simulink library "lib_all"  (Simulink 7.6)
settings: Solver=ode45  SolverMode=SingleTasking  StartTime=0.0  StopTime=10.0  RelTol=1e-3
values from neighbouring files (symbols this model uses):
  map_lib_03 = [6.8679 7.0159 7.4571 8.1849 9.1892 10.456 11.97 13.71 15.655 17.781 20.063 22.474 ... (803 elements, 73x11)]    [scr_simdata.mat]    (also in scr_simdata_all.mat)
  map_lib_06 = [2.5784 2.6727 2.9588 3.4453 4.1444 5.068 6.226 7.6242 9.2634 11.139 13.24 15.552 ... (803 elements, 73x11)]    [scr_simdata.mat]    (also in scr_simdata_all.mat)
  map_lib_09 = [0.0025784 0.0026727 0.0029588 0.0034453 0.0041444 0.005068 0.006226 0.0076242 0.0092634 0.011139 0.01324 0.015552 ... (803 elements, 73x11)]    [scr_simdata.mat]    (also in scr_simdata_all.mat)
  map_lib_10 = [2.74 2.75 2.76 2.77 2.78 2.79 2.8 2.81 2.82 2.83 2.84 2.85 ... (81 elements, 1x81)]    [scr_simdata.mat]    (also in scr_simdata_all.mat)
  map_lib_11 = [1.1983 1.2027 1.207 1.2113 1.2156 1.2198 1.2241 1.2283 1.2326 1.2368 1.241 1.2451 ... (81 elements, 1x81)]    [scr_simdata.mat]    (also in scr_simdata_all.mat)
  map_lib_12 = [2.74 2.75 2.76 2.77 2.78 2.79 2.8 2.81 2.82 2.83 2.84 2.85 ... (81 elements, 1x81)]    [scr_simdata.mat]    (also in scr_simdata_all.mat)
  map_lib_13 = [1.74 1.75 1.76 1.77 1.78 1.79 1.8 1.81 1.82 1.83 1.84 1.85 ... (81 elements, 1x81)]    [scr_simdata.mat]    (also in scr_simdata_all.mat)
  map_lib_16 = [0.00048828125 1.6159 1.8962 2.0821 2.2251 2.3426 2.4433 2.5318 2.611 2.683 2.749 2.8101 ... (1419 elements, 129x11)]    [scr_simdata.mat]    (also in scr_simdata_all.mat)
  map_mr2_01 = [0 800 1600 2400 3200 4000]    [scr_simdata.mat]    (also in scr_simdata_all.mat)
  map_mr2_02 = [1.32 1.32 1.33 1.34 1.36 1.38]    [scr_simdata.mat]    (also in scr_simdata_all.mat)
  map_mr2_03 = [0 800 1600 2400 3200 4000]    [scr_simdata.mat]    (also in scr_simdata_all.mat)
  map_mr2_04 = [1.32 1.32 1.33 1.34 1.36 1.38]    [scr_simdata.mat]    (also in scr_simdata_all.mat)
  map_mr2_05 = [0 800 1600 2400 3200 4000]    [scr_simdata.mat]    (also in scr_simdata_all.mat)
  map_mr2_06 = [1.32 1.32 1.33 1.34 1.36 1.38]    [scr_simdata.mat]    (also in scr_simdata_all.mat)
  Parameter1 = 6    [scr_simdata.mat]    (also in scr_simdata_all.mat)
  Parameter101 = 30    [scr_simdata.mat]    (also in scr_simdata_all.mat)
  Parameter102 = 12    [scr_simdata.mat]    (also in scr_simdata_all.mat)
  Parameter103 = -240    [scr_simdata.mat]    (also in scr_simdata_all.mat)
  Parameter108 = 48    [scr_simdata.mat]    (also in scr_simdata_all.mat)
  Parameter120 = 16    [scr_simdata.mat]    (also in scr_simdata_all.mat)
  Parameter121 = 87    [scr_simdata.mat]    (also in scr_simdata_all.mat)
  Parameter123 = 78    [scr_simdata.mat]    (also in scr_simdata_all.mat)
  Parameter124 = 60    [scr_simdata.mat]    (also in scr_simdata_all.mat)
  Parameter125 = -480    [scr_simdata.mat]    (also in scr_simdata_all.mat)
  Parameter130 = 96    [scr_simdata.mat]    (also in scr_simdata_all.mat)
  Parameter131 = 3    [scr_simdata.mat]    (also in scr_simdata_all.mat)
  Parameter144 = 3    [scr_simdata.mat]    (also in scr_simdata_all.mat)
  Parameter18 = 128    [scr_simdata.mat]    (also in scr_simdata_all.mat)
  Parameter19 = 16    [scr_simdata.mat]    (also in scr_simdata_all.mat)
  Parameter2 = 12    [scr_simdata.mat]    (also in scr_simdata_all.mat)
  Parameter20 = 7    [scr_simdata.mat]    (also in scr_simdata_all.mat)
  Parameter21 = 4    [scr_simdata.mat]    (also in scr_simdata_all.mat)
  Parameter3 = 18    [scr_simdata.mat]    (also in scr_simdata_all.mat)
  Parameter32 = 1    [scr_simdata.mat]    (also in scr_simdata_all.mat)
  Parameter34 = 24    [scr_simdata.mat]    (also in scr_simdata_all.mat)
  Parameter4 = 138    [scr_simdata.mat]    (also in scr_simdata_all.mat)
  Parameter42 = 48    [scr_simdata.mat]    (also in scr_simdata_all.mat)
  Parameter5 = 135    [scr_simdata.mat]    (also in scr_simdata_all.mat)
  Parameter55 = 0    [scr_simdata.mat]    (also in scr_simdata_all.mat)
  Parameter56 = 0    [scr_simdata.mat]    (also in scr_simdata_all.mat)
  Parameter57 = 1    [scr_simdata.mat]    (also in scr_simdata_all.mat)
  Parameter58 = 1    [scr_simdata.mat]    (also in scr_simdata_all.mat)
  Parameter59 = 2    [scr_simdata.mat]    (also in scr_simdata_all.mat)
  Parameter6 = 132    [scr_simdata.mat]    (also in scr_simdata_all.mat)
  Parameter60 = 2    [scr_simdata.mat]    (also in scr_simdata_all.mat)
  Parameter63 = 144    [scr_simdata.mat]    (also in scr_simdata_all.mat)
  Parameter7 = 126    [scr_simdata.mat]    (also in scr_simdata_all.mat)
  Parameter76 = 64    [scr_simdata.mat]    (also in scr_simdata_all.mat)
  Parameter77 = 135    [scr_simdata.mat]    (also in scr_simdata_all.mat)
  Parameter79 = 126    [scr_simdata.mat]    (also in scr_simdata_all.mat)
  Parameter80 = 108    [scr_simdata.mat]    (also in scr_simdata_all.mat)
  Parameter81 = 0    [scr_simdata.mat]    (also in scr_simdata_all.mat)
  Parameter86 = 0    [scr_simdata.mat]    (also in scr_simdata_all.mat)
  Parameter98 = 112    [scr_simdata.mat]    (also in scr_simdata_all.mat)
  Parameter99 = 39    [scr_simdata.mat]    (also in scr_simdata_all.mat)
undefined symbols (not defined in the file or in its folder): cylvolkp_hih_map2h, cylvolkp_map2h, pk1k_map2h
libraries: simulink x2
(part 12 of 36)

== system lib_all/SubSystem1540/SubSystem1549/SubSystem1554/SubSystem1555 ==
  Block4207 : Inport
  Block4208 : Inport  Port=2
  Block4209 : MultiPortSwitch  zeroidx=off  RndMeth=Zero  SaturateOnIntegerOverflow=on  InputSameDT=on
  SubSystem1556 : Subsystem  TreatAsAtomicUnit=on  mask(***ATC.DSRVEC***){bd_version=1.01.A, r0=DSM7, r1=-1, r2=, r3=, r4=, r5=, r6=, r7=, r8=, r9=, c0=, c1=, c2=, c3=, c4=, c5=, c6=, c7=, c8=, c9=}
      mask prompts: bd_version "Version"
      mask help: これはAutoCからのブロックです．編集禁止．
      settings: OpenFcn=warndlg('変更禁止!');
      mask Help: web(ui_blockhelpmng(gcb));
      mask Display: disp('ATC.DSRVEC')
  SubSystem1557 : Subsystem  TreatAsAtomicUnit=on  mask(***ATC.DSRVEC***){bd_version=1.01.A, r0=DSM6, r1=-1, r2=, r3=, r4=, r5=, r6=, r7=, r8=, r9=, c0=, c1=, c2=, c3=, c4=, c5=, c6=, c7=, c8=, c9=}
      mask prompts: bd_version "Version"
      mask help: これはAutoCからのブロックです．編集禁止．
      settings: OpenFcn=warndlg('変更禁止!');
      mask Help: web(ui_blockhelpmng(gcb));
      mask Display: disp('ATC.DSRVEC')
  SubSystem1558 : Subsystem  TreatAsAtomicUnit=on  mask(***ATC.DSRVEC***){bd_version=1.01.A, r0=DSM19, r1=-1, r2=, r3=, r4=, r5=, r6=, r7=, r8=, r9=, c0=, c1=, c2=, c3=, c4=, c5=, c6=, c7=, c8=, c9=}
      mask prompts: bd_version "Version"
      mask help: これはAutoCからのブロックです．編集禁止．
      settings: OpenFcn=warndlg('変更禁止!');
      mask Help: web(ui_blockhelpmng(gcb));
      mask Display: disp('ATC.DSRVEC')
  Block4210 : Outport
  wires:
  Block4207/1 -> Block4209/1
  Block4209/1 -> Block4210/1
  SubSystem1556/1(Output) -> Block4209/2
  SubSystem1557/1(Output) -> Block4209/3
  SubSystem1558/1(Output) -> Block4209/4
  Block4208/1 -> SubSystem1556/1(element), SubSystem1557/1(element), SubSystem1558/1(element)

== system lib_all/SubSystem1540/SubSystem1549/SubSystem1554/SubSystem1559 ==
  Block4211 : Inport
  Block4212 : Inport  Port=2
  Block4213 : MultiPortSwitch  zeroidx=off  RndMeth=Zero  SaturateOnIntegerOverflow=on  InputSameDT=on
  SubSystem1560 : Subsystem  TreatAsAtomicUnit=on  mask(***ATC.DSRVEC***){bd_version=1.01.A, r0=DSM7, r1=-1, r2=, r3=, r4=, r5=, r6=, r7=, r8=, r9=, c0=, c1=, c2=, c3=, c4=, c5=, c6=, c7=, c8=, c9=}
      mask prompts: bd_version "Version"
      mask help: これはAutoCからのブロックです．編集禁止．
      settings: OpenFcn=warndlg('変更禁止!');
      mask Help: web(ui_blockhelpmng(gcb));
      mask Display: disp('ATC.DSRVEC')
  SubSystem1561 : Subsystem  TreatAsAtomicUnit=on  mask(***ATC.DSRVEC***){bd_version=1.01.A, r0=DSM6, r1=-1, r2=, r3=, r4=, r5=, r6=, r7=, r8=, r9=, c0=, c1=, c2=, c3=, c4=, c5=, c6=, c7=, c8=, c9=}
      mask prompts: bd_version "Version"
      mask help: これはAutoCからのブロックです．編集禁止．
      settings: OpenFcn=warndlg('変更禁止!');
      mask Help: web(ui_blockhelpmng(gcb));
      mask Display: disp('ATC.DSRVEC')
  SubSystem1562 : Subsystem  TreatAsAtomicUnit=on  mask(***ATC.DSRVEC***){bd_version=1.01.A, r0=DSM19, r1=-1, r2=, r3=, r4=, r5=, r6=, r7=, r8=, r9=, c0=, c1=, c2=, c3=, c4=, c5=, c6=, c7=, c8=, c9=}
      mask prompts: bd_version "Version"
      mask help: これはAutoCからのブロックです．編集禁止．
      settings: OpenFcn=warndlg('変更禁止!');
      mask Help: web(ui_blockhelpmng(gcb));
      mask Display: disp('ATC.DSRVEC')
  Block4214 : Outport
  wires:
  Block4211/1 -> Block4213/1
  Block4213/1 -> Block4214/1
  SubSystem1560/1(Output) -> Block4213/2
  SubSystem1561/1(Output) -> Block4213/3
  SubSystem1562/1(Output) -> Block4213/4
  Block4212/1 -> SubSystem1560/1(element), SubSystem1561/1(element), SubSystem1562/1(element)

== system lib_all/SubSystem1540/SubSystem1549/SubSystem1563/SubSystem1564 ==
  note: ***編集禁止***
  element : Inport
  Inside_DSR : DataStoreRead  DataStoreName=DSM1
  Selector : Selector  IndexOptions=Index vector (port)  Indices=[1 3]  OutputSizes=1
  Output : Outport
  wires:
  Inside_DSR/1 -> Selector/1
  element/1 -> Selector/2
  Selector/1 -> Output/1

== system lib_all/SubSystem1540/SubSystem1549/SubSystem1563/SubSystem1565 ==
  Block4222 : Inport
  Block4223 : Inport  Port=2
  Block4224 : MultiPortSwitch  zeroidx=off  RndMeth=Zero  SaturateOnIntegerOverflow=on  InputSameDT=on
  SubSystem1566 : Subsystem  TreatAsAtomicUnit=on  mask(***ATC.DSRVEC***){bd_version=1.01.A, r0=DSM7, r1=-1, r2=, r3=, r4=, r5=, r6=, r7=, r8=, r9=, c0=, c1=, c2=, c3=, c4=, c5=, c6=, c7=, c8=, c9=}
      mask prompts: bd_version "Version"
      mask help: これはAutoCからのブロックです．編集禁止．
      settings: OpenFcn=warndlg('変更禁止!');
      mask Help: web(ui_blockhelpmng(gcb));
      mask Display: disp('ATC.DSRVEC')
  SubSystem1567 : Subsystem  TreatAsAtomicUnit=on  mask(***ATC.DSRVEC***){bd_version=1.01.A, r0=DSM6, r1=-1, r2=, r3=, r4=, r5=, r6=, r7=, r8=, r9=, c0=, c1=, c2=, c3=, c4=, c5=, c6=, c7=, c8=, c9=}
      mask prompts: bd_version "Version"
      mask help: これはAutoCからのブロックです．編集禁止．
      settings: OpenFcn=warndlg('変更禁止!');
      mask Help: web(ui_blockhelpmng(gcb));
      mask Display: disp('ATC.DSRVEC')
  SubSystem1568 : Subsystem  TreatAsAtomicUnit=on  mask(***ATC.DSRVEC***){bd_version=1.01.A, r0=DSM19, r1=-1, r2=, r3=, r4=, r5=, r6=, r7=, r8=, r9=, c0=, c1=, c2=, c3=, c4=, c5=, c6=, c7=, c8=, c9=}
      mask prompts: bd_version "Version"
      mask help: これはAutoCからのブロックです．編集禁止．
      settings: OpenFcn=warndlg('変更禁止!');
      mask Help: web(ui_blockhelpmng(gcb));
      mask Display: disp('ATC.DSRVEC')
  Block4225 : Outport
  wires:
  Block4222/1 -> Block4224/1
  Block4224/1 -> Block4225/1
  SubSystem1566/1(Output) -> Block4224/2
  SubSystem1567/1(Output) -> Block4224/3
  SubSystem1568/1(Output) -> Block4224/4
  Block4223/1 -> SubSystem1566/1(element), SubSystem1567/1(element), SubSystem1568/1(element)

== system lib_all/SubSystem1540/SubSystem1549/SubSystem1563/SubSystem1569 ==
  Block4226 : Inport
  Block4227 : Inport  Port=2
  Block4228 : MultiPortSwitch  zeroidx=off  RndMeth=Zero  SaturateOnIntegerOverflow=on  InputSameDT=on
  SubSystem1570 : Subsystem  TreatAsAtomicUnit=on  mask(***ATC.DSRVEC***){bd_version=1.01.A, r0=DSM7, r1=-1, r2=, r3=, r4=, r5=, r6=, r7=, r8=, r9=, c0=, c1=, c2=, c3=, c4=, c5=, c6=, c7=, c8=, c9=}
      mask prompts: bd_version "Version"
      mask help: これはAutoCからのブロックです．編集禁止．
      settings: OpenFcn=warndlg('変更禁止!');
      mask Help: web(ui_blockhelpmng(gcb));
      mask Display: disp('ATC.DSRVEC')
  SubSystem1571 : Subsystem  TreatAsAtomicUnit=on  mask(***ATC.DSRVEC***){bd_version=1.01.A, r0=DSM6, r1=-1, r2=, r3=, r4=, r5=, r6=, r7=, r8=, r9=, c0=, c1=, c2=, c3=, c4=, c5=, c6=, c7=, c8=, c9=}
      mask prompts: bd_version "Version"
      mask help: これはAutoCからのブロックです．編集禁止．
      settings: OpenFcn=warndlg('変更禁止!');
      mask Help: web(ui_blockhelpmng(gcb));
      mask Display: disp('ATC.DSRVEC')
  SubSystem1572 : Subsystem  TreatAsAtomicUnit=on  mask(***ATC.DSRVEC***){bd_version=1.01.A, r0=DSM19, r1=-1, r2=, r3=, r4=, r5=, r6=, r7=, r8=, r9=, c0=, c1=, c2=, c3=, c4=, c5=, c6=, c7=, c8=, c9=}
      mask prompts: bd_version "Version"
      mask help: これはAutoCからのブロックです．編集禁止．
      settings: OpenFcn=warndlg('変更禁止!');
      mask Help: web(ui_blockhelpmng(gcb));
      mask Display: disp('ATC.DSRVEC')
  Block4229 : Outport
  wires:
  Block4226/1 -> Block4228/1
  Block4228/1 -> Block4229/1
  SubSystem1570/1(Output) -> Block4228/2
  SubSystem1571/1(Output) -> Block4228/3
  SubSystem1572/1(Output) -> Block4228/4
  Block4227/1 -> SubSystem1570/1(element), SubSystem1571/1(element), SubSystem1572/1(element)

== system lib_all/SubSystem1540/SubSystem1549/SubSystem1573/SubSystem1574 ==
  note: ***編集禁止***
  element : Inport
  Inside_DSR : DataStoreRead  DataStoreName=DSM5
  Selector : Selector  IndexOptions=Index vector (port)  Indices=[1 3]  OutputSizes=1
  Output : Outport
  wires:
  Inside_DSR/1 -> Selector/1
  element/1 -> Selector/2
  Selector/1 -> Output/1

== system lib_all/SubSystem1540/SubSystem1549/SubSystem1573/SubSystem1575 ==
  Block4237 : Inport
  Block4238 : Inport  Port=2
  Block4239 : MultiPortSwitch  zeroidx=off  RndMeth=Zero  SaturateOnIntegerOverflow=on  InputSameDT=on
  SubSystem1576 : Subsystem  TreatAsAtomicUnit=on  mask(***ATC.DSRVEC***){bd_version=1.01.A, r0=DSM7, r1=-1, r2=, r3=, r4=, r5=, r6=, r7=, r8=, r9=, c0=, c1=, c2=, c3=, c4=, c5=, c6=, c7=, c8=, c9=}
      mask prompts: bd_version "Version"
      mask help: これはAutoCからのブロックです．編集禁止．
      settings: OpenFcn=warndlg('変更禁止!');
      mask Help: web(ui_blockhelpmng(gcb));
      mask Display: disp('ATC.DSRVEC')
  SubSystem1577 : Subsystem  TreatAsAtomicUnit=on  mask(***ATC.DSRVEC***){bd_version=1.01.A, r0=DSM6, r1=-1, r2=, r3=, r4=, r5=, r6=, r7=, r8=, r9=, c0=, c1=, c2=, c3=, c4=, c5=, c6=, c7=, c8=, c9=}
      mask prompts: bd_version "Version"
      mask help: これはAutoCからのブロックです．編集禁止．
      settings: OpenFcn=warndlg('変更禁止!');
      mask Help: web(ui_blockhelpmng(gcb));
      mask Display: disp('ATC.DSRVEC')
  SubSystem1578 : Subsystem  TreatAsAtomicUnit=on  mask(***ATC.DSRVEC***){bd_version=1.01.A, r0=DSM19, r1=-1, r2=, r3=, r4=, r5=, r6=, r7=, r8=, r9=, c0=, c1=, c2=, c3=, c4=, c5=, c6=, c7=, c8=, c9=}
      mask prompts: bd_version "Version"
      mask help: これはAutoCからのブロックです．編集禁止．
      settings: OpenFcn=warndlg('変更禁止!');
      mask Help: web(ui_blockhelpmng(gcb));
      mask Display: disp('ATC.DSRVEC')
  Block4240 : Outport
  wires:
  Block4237/1 -> Block4239/1
  Block4239/1 -> Block4240/1
  SubSystem1576/1(Output) -> Block4239/2
  SubSystem1577/1(Output) -> Block4239/3
  SubSystem1578/1(Output) -> Block4239/4
  Block4238/1 -> SubSystem1576/1(element), SubSystem1577/1(element), SubSystem1578/1(element)

== system lib_all/SubSystem1540/SubSystem1549/SubSystem1573/SubSystem1579 ==
  Block4241 : Inport
  Block4242 : Inport  Port=2
  Block4243 : MultiPortSwitch  zeroidx=off  RndMeth=Zero  SaturateOnIntegerOverflow=on  InputSameDT=on
  SubSystem1580 : Subsystem  TreatAsAtomicUnit=on  mask(***ATC.DSRVEC***){bd_version=1.01.A, r0=DSM7, r1=-1, r2=, r3=, r4=, r5=, r6=, r7=, r8=, r9=, c0=, c1=, c2=, c3=, c4=, c5=, c6=, c7=, c8=, c9=}
      mask prompts: bd_version "Version"
      mask help: これはAutoCからのブロックです．編集禁止．
      settings: OpenFcn=warndlg('変更禁止!');
      mask Help: web(ui_blockhelpmng(gcb));
      mask Display: disp('ATC.DSRVEC')
  SubSystem1581 : Subsystem  TreatAsAtomicUnit=on  mask(***ATC.DSRVEC***){bd_version=1.01.A, r0=DSM6, r1=-1, r2=, r3=, r4=, r5=, r6=, r7=, r8=, r9=, c0=, c1=, c2=, c3=, c4=, c5=, c6=, c7=, c8=, c9=}
      mask prompts: bd_version "Version"
      mask help: これはAutoCからのブロックです．編集禁止．
      settings: OpenFcn=warndlg('変更禁止!');
      mask Help: web(ui_blockhelpmng(gcb));
      mask Display: disp('ATC.DSRVEC')
  SubSystem1582 : Subsystem  TreatAsAtomicUnit=on  mask(***ATC.DSRVEC***){bd_version=1.01.A, r0=DSM19, r1=-1, r2=, r3=, r4=, r5=, r6=, r7=, r8=, r9=, c0=, c1=, c2=, c3=, c4=, c5=, c6=, c7=, c8=, c9=}
      mask prompts: bd_version "Version"
      mask help: これはAutoCからのブロックです．編集禁止．
      settings: OpenFcn=warndlg('変更禁止!');
      mask Help: web(ui_blockhelpmng(gcb));
      mask Display: disp('ATC.DSRVEC')
  Block4244 : Outport
  wires:
  Block4241/1 -> Block4243/1
  Block4243/1 -> Block4244/1
  SubSystem1580/1(Output) -> Block4243/2
  SubSystem1581/1(Output) -> Block4243/3
  SubSystem1582/1(Output) -> Block4243/4
  Block4242/1 -> SubSystem1580/1(element), SubSystem1581/1(element), SubSystem1582/1(element)

== system lib_all/SubSystem1540/SubSystem1549/SubSystem1583/SubSystem1584 ==
  note: ***編集禁止***
  element : Inport
  Inside_DSR : DataStoreRead  DataStoreName=DSM3
  Selector : Selector  IndexOptions=Index vector (port)  Indices=[1 3]  OutputSizes=1
  Output : Outport
  wires:
  Inside_DSR/1 -> Selector/1
  element/1 -> Selector/2
  Selector/1 -> Output/1

== system lib_all/SubSystem1540/SubSystem1549/SubSystem1583/SubSystem1585 ==
  Block4252 : Inport
  Block4253 : Inport  Port=2
  Block4254 : MultiPortSwitch  zeroidx=off  RndMeth=Zero  SaturateOnIntegerOverflow=on  InputSameDT=on
  SubSystem1586 : Subsystem  TreatAsAtomicUnit=on  mask(***ATC.DSRVEC***){bd_version=1.01.A, r0=DSM7, r1=-1, r2=, r3=, r4=, r5=, r6=, r7=, r8=, r9=, c0=, c1=, c2=, c3=, c4=, c5=, c6=, c7=, c8=, c9=}
      mask prompts: bd_version "Version"
      mask help: これはAutoCからのブロックです．編集禁止．
      settings: OpenFcn=warndlg('変更禁止!');
      mask Help: web(ui_blockhelpmng(gcb));
      mask Display: disp('ATC.DSRVEC')
  SubSystem1587 : Subsystem  TreatAsAtomicUnit=on  mask(***ATC.DSRVEC***){bd_version=1.01.A, r0=DSM6, r1=-1, r2=, r3=, r4=, r5=, r6=, r7=, r8=, r9=, c0=, c1=, c2=, c3=, c4=, c5=, c6=, c7=, c8=, c9=}
      mask prompts: bd_version "Version"
      mask help: これはAutoCからのブロックです．編集禁止．
      settings: OpenFcn=warndlg('変更禁止!');
      mask Help: web(ui_blockhelpmng(gcb));
      mask Display: disp('ATC.DSRVEC')
  SubSystem1588 : Subsystem  TreatAsAtomicUnit=on  mask(***ATC.DSRVEC***){bd_version=1.01.A, r0=DSM19, r1=-1, r2=, r3=, r4=, r5=, r6=, r7=, r8=, r9=, c0=, c1=, c2=, c3=, c4=, c5=, c6=, c7=, c8=, c9=}
      mask prompts: bd_version "Version"
      mask help: これはAutoCからのブロックです．編集禁止．
      settings: OpenFcn=warndlg('変更禁止!');
      mask Help: web(ui_blockhelpmng(gcb));
      mask Display: disp('ATC.DSRVEC')
  Block4255 : Outport
  wires:
  Block4252/1 -> Block4254/1
  Block4254/1 -> Block4255/1
  SubSystem1586/1(Output) -> Block4254/2
  SubSystem1587/1(Output) -> Block4254/3
  SubSystem1588/1(Output) -> Block4254/4
  Block4253/1 -> SubSystem1586/1(element), SubSystem1587/1(element), SubSystem1588/1(element)

== system lib_all/SubSystem1540/SubSystem1549/SubSystem1583/SubSystem1589 ==
  Block4256 : Inport
  Block4257 : Inport  Port=2
  Block4258 : MultiPortSwitch  zeroidx=off  RndMeth=Zero  SaturateOnIntegerOverflow=on  InputSameDT=on
  SubSystem1590 : Subsystem  TreatAsAtomicUnit=on  mask(***ATC.DSRVEC***){bd_version=1.01.A, r0=DSM7, r1=-1, r2=, r3=, r4=, r5=, r6=, r7=, r8=, r9=, c0=, c1=, c2=, c3=, c4=, c5=, c6=, c7=, c8=, c9=}
      mask prompts: bd_version "Version"
      mask help: これはAutoCからのブロックです．編集禁止．
      settings: OpenFcn=warndlg('変更禁止!');
      mask Help: web(ui_blockhelpmng(gcb));
      mask Display: disp('ATC.DSRVEC')
  SubSystem1591 : Subsystem  TreatAsAtomicUnit=on  mask(***ATC.DSRVEC***){bd_version=1.01.A, r0=DSM6, r1=-1, r2=, r3=, r4=, r5=, r6=, r7=, r8=, r9=, c0=, c1=, c2=, c3=, c4=, c5=, c6=, c7=, c8=, c9=}
      mask prompts: bd_version "Version"
      mask help: これはAutoCからのブロックです．編集禁止．
      settings: OpenFcn=warndlg('変更禁止!');
      mask Help: web(ui_blockhelpmng(gcb));
      mask Display: disp('ATC.DSRVEC')
  SubSystem1592 : Subsystem  TreatAsAtomicUnit=on  mask(***ATC.DSRVEC***){bd_version=1.01.A, r0=DSM19, r1=-1, r2=, r3=, r4=, r5=, r6=, r7=, r8=, r9=, c0=, c1=, c2=, c3=, c4=, c5=, c6=, c7=, c8=, c9=}
      mask prompts: bd_version "Version"
      mask help: これはAutoCからのブロックです．編集禁止．
      settings: OpenFcn=warndlg('変更禁止!');
      mask Help: web(ui_blockhelpmng(gcb));
      mask Display: disp('ATC.DSRVEC')
  Block4259 : Outport
  wires:
  Block4256/1 -> Block4258/1
  Block4258/1 -> Block4259/1
  SubSystem1590/1(Output) -> Block4258/2
  SubSystem1591/1(Output) -> Block4258/3
  SubSystem1592/1(Output) -> Block4258/4
  Block4257/1 -> SubSystem1590/1(element), SubSystem1591/1(element), SubSystem1592/1(element)

== system lib_all/SubSystem1593/SubSystem1595/SubSystem1598/SubSystem1599 ==
  note: ***編集禁止***
  mult : Inport
  div : Inport  Port=2
  init : Inport  Port=3
  Product : Subsystem  TreatAsAtomicUnit=on
      settings: RTWSystemCode=Inline
  Out : Outport
  wires:
  Product/1(Out) -> Out/1
  mult/1 -> Product/1(mult)
  div/1 -> Product/2(div)
  init/1 -> Product/3(init)

== system lib_all/SubSystem1593/SubSystem1595/SubSystem1598/SubSystem1600 ==
  note: ***編集禁止***
  element : Inport
  Inside_DSR : DataStoreRead  DataStoreName=DSM95
  Selector : Selector  IndexOptions=Index vector (port)  Indices=[1 3]  OutputSizes=1
  Output : Outport
  wires:
  Selector/1 -> Output/1
  element/1 -> Selector/2
  Inside_DSR/1 -> Selector/1

== system lib_all/SubSystem1593/SubSystem1595/SubSystem1598/SubSystem1601 ==
  Block4300 : Inport
  Block4301 : Inport  Port=2
  Block4302 : Inport  Port=3
  Block4303 : Sum  OutDataTypeStr=double  RndMeth=Zero  SaturateOnIntegerOverflow=on  InputSameDT=on
  Block4304 : Sum  Inputs=+-  OutDataTypeStr=double  RndMeth=Zero  SaturateOnIntegerOverflow=on  InputSameDT=on
  Block4305 : Switch  Threshold=0.5  RndMeth=Zero  SaturateOnIntegerOverflow=on  InputSameDT=on
  Block4306 : Switch  Threshold=0.5  RndMeth=Zero  SaturateOnIntegerOverflow=on  InputSameDT=on
  SubSystem1602 : Subsystem
  SubSystem1603 : Subsystem
  Block4307 : Outport
  wires:
  Block4301/1 -> SubSystem1602/1(In1), Block4304/1, SubSystem1603/1(In1), Block4306/3, Block4303/2
  Block4300/1 -> Block4303/1, Block4304/2, SubSystem1602/2(In2)
  SubSystem1602/1(Out1) -> Block4305/2
  Block4302/1 -> SubSystem1603/2(In2)
  SubSystem1603/1(Out1) -> Block4306/2
  Block4306/1 -> Block4305/3
  Block4303/1 -> Block4306/1
  Block4305/1 -> Block4307/1
  Block4304/1 -> Block4305/1

== system lib_all/SubSystem1593/SubSystem1595/SubSystem1604/SubSystem1605 ==
  note: ***編集禁止***
  In1 : Inport
  In2 : Inport  Port=2
  LO1 : Logic
  RO1 : RelationalOperator  Operator=<  InputSameDT=on
  RO2 : RelationalOperator  Operator=~=  InputSameDT=on
  single1 : DataTypeConversion  OutDataTypeStr=single  SaturateOnIntegerOverflow=on  RndMeth=Zero
  single2 : DataTypeConversion  OutDataTypeStr=single  SaturateOnIntegerOverflow=on  RndMeth=Zero
  Out1 : Outport
  wires:
  LO1/1 -> Out1/1
  RO2/1 -> LO1/2
  RO1/1 -> LO1/1
  In2/1 -> single2/1
  In1/1 -> single1/1
  single1/1 -> RO2/1, RO1/1
  single2/1 -> RO2/2, RO1/2

== system lib_all/SubSystem1593/SubSystem1595/SubSystem1604/SubSystem1606 ==
  note: ***編集禁止***
  In1 : Inport
  In2 : Inport  Port=2
  RO1 : RelationalOperator  Operator===  InputSameDT=on
  single1 : DataTypeConversion  OutDataTypeStr=single  SaturateOnIntegerOverflow=on  RndMeth=Zero
  single2 : DataTypeConversion  OutDataTypeStr=single  SaturateOnIntegerOverflow=on  RndMeth=Zero
  Out1 : Outport
  wires:
  RO1/1 -> Out1/1
  In2/1 -> single2/1
  In1/1 -> single1/1
  single1/1 -> RO1/1
  single2/1 -> RO1/2

== system lib_all/SubSystem1593/SubSystem1595/SubSystem1604/SubSystem1607 ==
  note: ***編集禁止***
  In1 : Inport
  In2 : Inport  Port=2
  RO1 : RelationalOperator  Operator===  InputSameDT=on
  single1 : DataTypeConversion  OutDataTypeStr=single  SaturateOnIntegerOverflow=on  RndMeth=Zero
  single2 : DataTypeConversion  OutDataTypeStr=single  SaturateOnIntegerOverflow=on  RndMeth=Zero
  Out1 : Outport
  wires:
  RO1/1 -> Out1/1
  In2/1 -> single2/1
  In1/1 -> single1/1
  single1/1 -> RO1/1
  single2/1 -> RO1/2

== system lib_all/SubSystem1593/SubSystem1595/SubSystem1604/SubSystem1608 ==
  note: ***編集禁止***
  In1 : Inport
  In2 : Inport  Port=2
  RO1 : RelationalOperator  Operator===  InputSameDT=on
  single1 : DataTypeConversion  OutDataTypeStr=single  SaturateOnIntegerOverflow=on  RndMeth=Zero
  single2 : DataTypeConversion  OutDataTypeStr=single  SaturateOnIntegerOverflow=on  RndMeth=Zero
  Out1 : Outport
  wires:
  RO1/1 -> Out1/1
  In2/1 -> single2/1
  In1/1 -> single1/1
  single1/1 -> RO1/1
  single2/1 -> RO1/2

== system lib_all/SubSystem1593/SubSystem1595/SubSystem1604/SubSystem1609 ==
  Block4330 : Inport
  Block4331 : Inport  Port=2
  Block4332 : EnablePort
  Block4333 : Demux  Outputs=2
  Block4334 : Mux  Inputs=2
  SubSystem1610 : Subsystem
  SubSystem1614 : Subsystem
  Block4343 : Outport
  wires:
  Block4330/1 -> Block4333/1
  Block4331/1 -> SubSystem1614/1(Block4339), SubSystem1610/1(Block4335)
  Block4333/1 -> SubSystem1610/2(Block4336)
  Block4333/2 -> SubSystem1614/2(Block4340)
  SubSystem1610/1(Block4338) -> Block4334/1
  SubSystem1614/1(Block4342) -> Block4334/2
  Block4334/1 -> Block4343/1

== system lib_all/SubSystem1593/SubSystem1595/SubSystem1604/SubSystem1618 ==
  Block4344 : Inport
  Block4345 : Inport  Port=2
  Block4346 : EnablePort
  Block4347 : Demux  Outputs=2
  Block4348 : Mux  Inputs=2
  Block4349 : Sum  OutDataTypeStr=double  RndMeth=Zero  SaturateOnIntegerOverflow=on  InputSameDT=on
  SubSystem1619 : Subsystem  TreatAsAtomicUnit=on  mask(***ATC.DSRVEC***){bd_version=1.01.A, r0=DSM1, r1=-1, r2=, r3=, r4=, r5=, r6=, r7=, r8=, r9=, c0=, c1=, c2=, c3=, c4=, c5=, c6=, c7=, c8=, c9=}
      mask prompts: bd_version "Version"
      mask help: これはAutoCからのブロックです．編集禁止．
      settings: OpenFcn=warndlg('変更禁止!');
      mask Help: web(ui_blockhelpmng(gcb));
      mask Display: disp('ATC.DSRVEC')
  SubSystem1620 : Subsystem
  SubSystem1624 : Subsystem
  Block4358 : Outport
  wires:
  Block4344/1 -> Block4347/1
  Block4347/1 -> SubSystem1620/2(Block4351)
  Block4347/2 -> SubSystem1624/2(Block4355)
  SubSystem1620/1(Block4353) -> Block4348/1
  SubSystem1624/1(Block4357) -> Block4348/2
  Block4349/1 -> Block4358/1
  SubSystem1619/1(Output) -> Block4349/1
  Block4348/1 -> Block4349/2
  Block4345/1 -> SubSystem1624/1(Block4354), SubSystem1620/1(Block4350), SubSystem1619/1(element)

== system lib_all/SubSystem1593/SubSystem1595/SubSystem1604/SubSystem1628 ==
  Block4359 : Inport
  Block4360 : Inport  Port=2
  Block4361 : EnablePort
  Block4362 : Demux  Outputs=2
  Block4363 : Mux  Inputs=2
  Block4364 : Sum  OutDataTypeStr=double  RndMeth=Zero  SaturateOnIntegerOverflow=on  InputSameDT=on
  SubSystem1629 : Subsystem  TreatAsAtomicUnit=on  mask(***ATC.DSRVEC***){bd_version=1.01.A, r0=DSM5, r1=-1, r2=, r3=, r4=, r5=, r6=, r7=, r8=, r9=, c0=, c1=, c2=, c3=, c4=, c5=, c6=, c7=, c8=, c9=}
      mask prompts: bd_version "Version"
      mask help: これはAutoCからのブロックです．編集禁止．
      settings: OpenFcn=warndlg('変更禁止!');
      mask Help: web(ui_blockhelpmng(gcb));
      mask Display: disp('ATC.DSRVEC')
  SubSystem1630 : Subsystem
  SubSystem1634 : Subsystem
  Block4373 : Outport
  wires:
  Block4359/1 -> Block4362/1
  Block4362/1 -> SubSystem1630/2(Block4366)
  Block4362/2 -> SubSystem1634/2(Block4370)
  SubSystem1630/1(Block4368) -> Block4363/1
  SubSystem1634/1(Block4372) -> Block4363/2
  Block4364/1 -> Block4373/1
  SubSystem1629/1(Output) -> Block4364/1
  Block4363/1 -> Block4364/2
  Block4360/1 -> SubSystem1634/1(Block4369), SubSystem1630/1(Block4365), SubSystem1629/1(element)

== system lib_all/SubSystem1593/SubSystem1595/SubSystem1604/SubSystem1638 ==
  Block4374 : Inport
  Block4375 : Inport  Port=2
  Block4376 : EnablePort
  Block4377 : Demux  Outputs=2
  Block4378 : Mux  Inputs=2
  Block4379 : Sum  OutDataTypeStr=double  RndMeth=Zero  SaturateOnIntegerOverflow=on  InputSameDT=on
  SubSystem1639 : Subsystem  TreatAsAtomicUnit=on  mask(***ATC.DSRVEC***){bd_version=1.01.A, r0=DSM3, r1=-1, r2=, r3=, r4=, r5=, r6=, r7=, r8=, r9=, c0=, c1=, c2=, c3=, c4=, c5=, c6=, c7=, c8=, c9=}
      mask prompts: bd_version "Version"
      mask help: これはAutoCからのブロックです．編集禁止．
      settings: OpenFcn=warndlg('変更禁止!');
      mask Help: web(ui_blockhelpmng(gcb));
      mask Display: disp('ATC.DSRVEC')
  SubSystem1640 : Subsystem
  SubSystem1644 : Subsystem
  Block4388 : Outport
  wires:
  Block4378/1 -> Block4379/2
  SubSystem1639/1(Output) -> Block4379/1
  Block4379/1 -> Block4388/1
  SubSystem1644/1(Block4387) -> Block4378/2
  SubSystem1640/1(Block4383) -> Block4378/1
  Block4377/2 -> SubSystem1644/2(Block4385)
  Block4377/1 -> SubSystem1640/2(Block4381)
  Block4374/1 -> Block4377/1
  Block4375/1 -> SubSystem1644/1(Block4384), SubSystem1640/1(Block4380), SubSystem1639/1(element)

== system lib_all/SubSystem1593/SubSystem1648/SubSystem1650/SubSystem1651 ==
  Block4403 : Inport
  Block4404 : Inport  Port=2
  Block4405 : Constant  Value=Parameter32  OutDataTypeStr=double  SampleTime=-1
  Block4406 : Constant  Value=2  OutDataTypeStr=double  SampleTime=-1
  Block4407 : Constant  Value=Parameter42  OutDataTypeStr=double  SampleTime=-1
  Block4408 : Constant  Value=0  OutDataTypeStr=double  SampleTime=-1
  Block4409 : Constant  Value=72  OutDataTypeStr=double  SampleTime=-1
  Block4410 : Constant  Value=Parameter34  OutDataTypeStr=double  SampleTime=-1
  Block4411 : Logic  Operator=OR
  Block4412 : Sum  Inputs=+-  OutDataTypeStr=double  RndMeth=Zero  SaturateOnIntegerOverflow=on  InputSameDT=on
  Block4413 : Switch  Threshold=0.5  RndMeth=Zero  SaturateOnIntegerOverflow=on  InputSameDT=on
  Block4414 : Switch  Threshold=0.5  RndMeth=Zero  SaturateOnIntegerOverflow=on  InputSameDT=on
  SubSystem1652 : Subsystem
  SubSystem1653 : Subsystem
  SubSystem1654 : Subsystem
      settings: RTWSystemCode=Inline
  Block4421 : Outport
  wires:
  Block4413/1 -> Block4412/2
  Block4410/1 -> Block4413/3
  Block4407/1 -> Block4413/1
  Block4411/1 -> Block4414/2
  SubSystem1653/1(Out1) -> Block4411/2
  SubSystem1652/1(Out1) -> Block4413/2, Block4411/1
  Block4406/1 -> SubSystem1653/2(In2)
  Block4405/1 -> SubSystem1652/1(In1)
  Block4404/1 -> SubSystem1653/1(In1), SubSystem1652/2(In2)
  Block4403/1 -> Block4412/1, Block4414/3
  Block4412/1 -> SubSystem1654/2(Block4416)
  Block4408/1 -> SubSystem1654/3(Block4417)
  Block4409/1 -> SubSystem1654/1(Block4415)
  SubSystem1654/1(Block4420) -> Block4414/1
  Block4414/1 -> Block4421/1

== system lib_all/SubSystem1593/SubSystem1648/SubSystem1650/SubSystem1656 ==
  Block4422 : Inport
  Block4423 : Inport  Port=2
  Block4424 : Inport  Port=3
  Block4425 : Sum  OutDataTypeStr=double  RndMeth=Zero  SaturateOnIntegerOverflow=on  InputSameDT=on
  Block4426 : Switch  Threshold=0.5  RndMeth=Zero  SaturateOnIntegerOverflow=on  InputSameDT=on
  SubSystem1657 : Subsystem
  Block4427 : Outport
  wires:
  Block4422/1 -> Block4425/1
  Block4426/1 -> Block4427/1
  Block4425/1 -> Block4426/1
  SubSystem1657/1(Out1) -> Block4426/2
  Block4424/1 -> SubSystem1657/2(In2)
  Block4423/1 -> Block4425/2, Block4426/3, SubSystem1657/1(In1)

== system lib_all/SubSystem1593/SubSystem1648/SubSystem1658/SubSystem1659 ==
  note: ***編集禁止***
  In1 : Inport
  In2 : Inport  Port=2
  RO1 : RelationalOperator  Operator=>  InputSameDT=on
  single1 : DataTypeConversion  OutDataTypeStr=single  SaturateOnIntegerOverflow=on  RndMeth=Zero
  single2 : DataTypeConversion  OutDataTypeStr=single  SaturateOnIntegerOverflow=on  RndMeth=Zero
  Out1 : Outport
  wires:
  RO1/1 -> Out1/1
  In2/1 -> single2/1
  In1/1 -> single1/1
  single1/1 -> RO1/1
  single2/1 -> RO1/2

== system lib_all/SubSystem1662/SubSystem1664/SubSystem1665/SubSystem1666 ==
  note: ***編集禁止***
  In1 : Inport
  In2 : Inport  Port=2
  RO1 : RelationalOperator  Operator===  InputSameDT=on
  single1 : DataTypeConversion  OutDataTypeStr=single  SaturateOnIntegerOverflow=on  RndMeth=Zero
  single2 : DataTypeConversion  OutDataTypeStr=single  SaturateOnIntegerOverflow=on  RndMeth=Zero
  Out1 : Outport
  wires:
  RO1/1 -> Out1/1
  In2/1 -> single2/1
  In1/1 -> single1/1
  single1/1 -> RO1/1
  single2/1 -> RO1/2

== system lib_all/SubSystem1662/SubSystem1664/SubSystem1665/SubSystem1667 ==
  note: ***編集禁止***
  In1 : Inport
  In2 : Inport  Port=2
  RO1 : RelationalOperator  Operator===  InputSameDT=on
  single1 : DataTypeConversion  OutDataTypeStr=single  SaturateOnIntegerOverflow=on  RndMeth=Zero
  single2 : DataTypeConversion  OutDataTypeStr=single  SaturateOnIntegerOverflow=on  RndMeth=Zero
  Out1 : Outport
  wires:
  RO1/1 -> Out1/1
  In2/1 -> single2/1
  In1/1 -> single1/1
  single1/1 -> RO1/1
  single2/1 -> RO1/2

== system lib_all/SubSystem1662/SubSystem1664/SubSystem1665/SubSystem1668 ==
  Block4477 : Inport
  Block4478 : Inport  Port=2
  Block4479 : Inport  Port=3
  Block4480 : Sum  OutDataTypeStr=double  RndMeth=Zero  SaturateOnIntegerOverflow=on  InputSameDT=on
  Block4481 : Switch  Threshold=0.5  RndMeth=Zero  SaturateOnIntegerOverflow=on  InputSameDT=on
  SubSystem1669 : Subsystem
  Block4482 : Outport
  wires:
  Block4477/1 -> Block4480/1
  Block4481/1 -> Block4482/1
  Block4480/1 -> Block4481/1
  SubSystem1669/1(Out1) -> Block4481/2
  Block4479/1 -> SubSystem1669/2(In2)
  Block4478/1 -> Block4480/2, Block4481/3, SubSystem1669/1(In1)

== system lib_all/SubSystem1662/SubSystem1664/SubSystem1670/SubSystem1671 ==
  note: ***編集禁止***
  In1 : Inport
  In2 : Inport  Port=2
  LO1 : Logic
  RO1 : RelationalOperator  Operator=<  InputSameDT=on
  RO2 : RelationalOperator  Operator=~=  InputSameDT=on
  single1 : DataTypeConversion  OutDataTypeStr=single  SaturateOnIntegerOverflow=on  RndMeth=Zero
  single2 : DataTypeConversion  OutDataTypeStr=single  SaturateOnIntegerOverflow=on  RndMeth=Zero
  Out1 : Outport
  wires:
  In2/1 -> single2/1
  In1/1 -> single1/1
  single1/1 -> RO1/1, RO2/1
  single2/1 -> RO1/2, RO2/2
  RO1/1 -> LO1/1
  RO2/1 -> LO1/2
  LO1/1 -> Out1/1

== system lib_all/SubSystem1674/SubSystem1687/SubSystem1690/SubSystem1691 ==
  Block4582 : Inport
  Block4583 : Inport  Port=2
  Block4584 : Constant  Value=Parameter32  OutDataTypeStr=double  SampleTime=-1
  Block4585 : Constant  Value=2  OutDataTypeStr=double  SampleTime=-1
  Block4586 : Constant  Value=Parameter42  OutDataTypeStr=double  SampleTime=-1
  Block4587 : Constant  Value=0  OutDataTypeStr=double  SampleTime=-1
  Block4588 : Constant  Value=72  OutDataTypeStr=double  SampleTime=-1
  Block4589 : Constant  Value=Parameter34  OutDataTypeStr=double  SampleTime=-1
  Block4590 : Logic  Operator=OR
  Block4591 : Sum  Inputs=+-  OutDataTypeStr=double  RndMeth=Zero  SaturateOnIntegerOverflow=on  InputSameDT=on
  Block4592 : Switch  Threshold=0.5  RndMeth=Zero  SaturateOnIntegerOverflow=on  InputSameDT=on
  Block4593 : Switch  Threshold=0.5  RndMeth=Zero  SaturateOnIntegerOverflow=on  InputSameDT=on
  SubSystem1692 : Subsystem
  SubSystem1693 : Subsystem
  SubSystem1694 : Subsystem
      settings: RTWSystemCode=Inline
  Block4600 : Outport
  wires:
  SubSystem1694/1(Block4599) -> Block4593/1
  Block4588/1 -> SubSystem1694/1(Block4594)
  Block4587/1 -> SubSystem1694/3(Block4596)
  Block4591/1 -> SubSystem1694/2(Block4595)
  Block4582/1 -> Block4593/3, Block4591/1
  Block4583/1 -> SubSystem1692/2(In2), SubSystem1693/1(In1)
  Block4584/1 -> SubSystem1692/1(In1)
  Block4585/1 -> SubSystem1693/2(In2)
  SubSystem1692/1(Out1) -> Block4590/1, Block4592/2
  SubSystem1693/1(Out1) -> Block4590/2
  Block4590/1 -> Block4593/2
  Block4586/1 -> Block4592/1
  Block4589/1 -> Block4592/3
  Block4592/1 -> Block4591/2
  Block4593/1 -> Block4600/1

== system lib_all/SubSystem1674/SubSystem1687/SubSystem1690/SubSystem1696 ==
  Block4601 : Inport
  Block4602 : Inport  Port=2
  Block4603 : Inport  Port=3
  Block4604 : Sum  OutDataTypeStr=double  RndMeth=Zero  SaturateOnIntegerOverflow=on  InputSameDT=on
  Block4605 : Switch  Threshold=0.5  RndMeth=Zero  SaturateOnIntegerOverflow=on  InputSameDT=on
  SubSystem1697 : Subsystem
  Block4606 : Outport
  wires:
  Block4602/1 -> SubSystem1697/1(In1), Block4605/3, Block4604/2
  Block4603/1 -> SubSystem1697/2(In2)
  SubSystem1697/1(Out1) -> Block4605/2
  Block4604/1 -> Block4605/1
  Block4605/1 -> Block4606/1
  Block4601/1 -> Block4604/1

== system lib_all/SubSystem1674/SubSystem1687/SubSystem1699/SubSystem1700 ==
  note: ***編集禁止***
  In1 : Inport
  In2 : Inport  Port=2
  RO1 : RelationalOperator  Operator=>  InputSameDT=on
  single1 : DataTypeConversion  OutDataTypeStr=single  SaturateOnIntegerOverflow=on  RndMeth=Zero
  single2 : DataTypeConversion  OutDataTypeStr=single  SaturateOnIntegerOverflow=on  RndMeth=Zero
  Out1 : Outport
  wires:
  single2/1 -> RO1/2
  single1/1 -> RO1/1
  In1/1 -> single1/1
  In2/1 -> single2/1
  RO1/1 -> Out1/1

== system lib_all/SubSystem1674/SubSystem1701/SubSystem1703/SubSystem1704 ==
  note: ***編集禁止***
  mult : Inport
  div : Inport  Port=2
  init : Inport  Port=3
  Product : Subsystem  TreatAsAtomicUnit=on
      settings: RTWSystemCode=Inline
  Out : Outport
  wires:
  init/1 -> Product/3(init)
  div/1 -> Product/2(div)
  mult/1 -> Product/1(mult)
  Product/1(Out) -> Out/1

== system lib_all/SubSystem1674/SubSystem1701/SubSystem1703/SubSystem1705 ==
  note: ***編集禁止***
  element : Inport
  Inside_DSR : DataStoreRead  DataStoreName=DSM95
  Selector : Selector  IndexOptions=Index vector (port)  Indices=[1 3]  OutputSizes=1
  Output : Outport
  wires:
  Inside_DSR/1 -> Selector/1
  element/1 -> Selector/2
  Selector/1 -> Output/1

== system lib_all/SubSystem1674/SubSystem1701/SubSystem1703/SubSystem1706 ==
  Block4649 : Inport
  Block4650 : Inport  Port=2
  Block4651 : Inport  Port=3
  Block4652 : Sum  OutDataTypeStr=double  RndMeth=Zero  SaturateOnIntegerOverflow=on  InputSameDT=on
  Block4653 : Sum  Inputs=+-  OutDataTypeStr=double  RndMeth=Zero  SaturateOnIntegerOverflow=on  InputSameDT=on
  Block4654 : Switch  Threshold=0.5  RndMeth=Zero  SaturateOnIntegerOverflow=on  InputSameDT=on
  Block4655 : Switch  Threshold=0.5  RndMeth=Zero  SaturateOnIntegerOverflow=on  InputSameDT=on
  SubSystem1707 : Subsystem
  SubSystem1708 : Subsystem
  Block4656 : Outport
  wires:
  Block4653/1 -> Block4654/1
  Block4654/1 -> Block4656/1
  Block4652/1 -> Block4655/1
  Block4655/1 -> Block4654/3
  SubSystem1708/1(Out1) -> Block4655/2
  Block4651/1 -> SubSystem1708/2(In2)
  SubSystem1707/1(Out1) -> Block4654/2
  Block4649/1 -> SubSystem1707/2(In2), Block4653/2, Block4652/1
  Block4650/1 -> Block4652/2, Block4655/3, SubSystem1708/1(In1), Block4653/1, SubSystem1707/1(In1)

== system lib_all/SubSystem1674/SubSystem1701/SubSystem1709/SubSystem1710 ==
  note: ***編集禁止***
  In1 : Inport
  In2 : Inport  Port=2
  LO1 : Logic
  RO1 : RelationalOperator  Operator=<  InputSameDT=on
  RO2 : RelationalOperator  Operator=~=  InputSameDT=on
  single1 : DataTypeConversion  OutDataTypeStr=single  SaturateOnIntegerOverflow=on  RndMeth=Zero
  single2 : DataTypeConversion  OutDataTypeStr=single  SaturateOnIntegerOverflow=on  RndMeth=Zero
  Out1 : Outport
  wires:
  single2/1 -> RO1/2, RO2/2
  single1/1 -> RO1/1, RO2/1
  In1/1 -> single1/1
  In2/1 -> single2/1
  RO1/1 -> LO1/1
  RO2/1 -> LO1/2
  LO1/1 -> Out1/1

== system lib_all/SubSystem1674/SubSystem1701/SubSystem1709/SubSystem1711 ==
  note: ***編集禁止***
  In1 : Inport
  In2 : Inport  Port=2
  RO1 : RelationalOperator  Operator===  InputSameDT=on
  single1 : DataTypeConversion  OutDataTypeStr=single  SaturateOnIntegerOverflow=on  RndMeth=Zero
  single2 : DataTypeConversion  OutDataTypeStr=single  SaturateOnIntegerOverflow=on  RndMeth=Zero
  Out1 : Outport
  wires:
  single2/1 -> RO1/2
  single1/1 -> RO1/1
  In1/1 -> single1/1
  In2/1 -> single2/1
  RO1/1 -> Out1/1

== system lib_all/SubSystem1674/SubSystem1701/SubSystem1709/SubSystem1712 ==
  note: ***編集禁止***
  In1 : Inport
  In2 : Inport  Port=2
  RO1 : RelationalOperator  Operator===  InputSameDT=on
  single1 : DataTypeConversion  OutDataTypeStr=single  SaturateOnIntegerOverflow=on  RndMeth=Zero
  single2 : DataTypeConversion  OutDataTypeStr=single  SaturateOnIntegerOverflow=on  RndMeth=Zero
  Out1 : Outport
  wires:
  single2/1 -> RO1/2
  single1/1 -> RO1/1
  In1/1 -> single1/1
  In2/1 -> single2/1
  RO1/1 -> Out1/1

== system lib_all/SubSystem1674/SubSystem1701/SubSystem1709/SubSystem1713 ==
  Block4677 : Inport
  Block4678 : Inport  Port=2
  Block4679 : EnablePort
  Block4680 : Demux  Outputs=2
  Block4681 : Mux  Inputs=2
  SubSystem1714 : Subsystem
  SubSystem1718 : Subsystem
  Block4690 : Outport
  wires:
  Block4681/1 -> Block4690/1
  SubSystem1718/1(Block4689) -> Block4681/2
  SubSystem1714/1(Block4685) -> Block4681/1
  Block4680/2 -> SubSystem1718/2(Block4687)
  Block4680/1 -> SubSystem1714/2(Block4683)
  Block4678/1 -> SubSystem1714/1(Block4682), SubSystem1718/1(Block4686)
  Block4677/1 -> Block4680/1

== system lib_all/SubSystem1674/SubSystem1701/SubSystem1709/SubSystem1722 ==
  Block4691 : Inport
  Block4692 : Inport  Port=2
  Block4693 : EnablePort
  Block4694 : Demux  Outputs=2
  Block4695 : Mux  Inputs=2
  Block4696 : Sum  OutDataTypeStr=double  RndMeth=Zero  SaturateOnIntegerOverflow=on  InputSameDT=on
  SubSystem1723 : Subsystem  TreatAsAtomicUnit=on  mask(***ATC.DSRVEC***){bd_version=1.01.A, r0=DSM4, r1=-1, r2=, r3=, r4=, r5=, r6=, r7=, r8=, r9=, c0=, c1=, c2=, c3=, c4=, c5=, c6=, c7=, c8=, c9=}
      mask prompts: bd_version "Version"
      mask help: これはAutoCからのブロックです．編集禁止．
      settings: OpenFcn=warndlg('変更禁止!');
      mask Help: web(ui_blockhelpmng(gcb));
      mask Display: disp('ATC.DSRVEC')
  SubSystem1724 : Subsystem
  SubSystem1728 : Subsystem
  Block4705 : Outport
  wires:
  Block4692/1 -> SubSystem1723/1(element), SubSystem1724/1(Block4697), SubSystem1728/1(Block4701)
  Block4695/1 -> Block4696/2
  SubSystem1723/1(Output) -> Block4696/1
  Block4696/1 -> Block4705/1
  SubSystem1728/1(Block4704) -> Block4695/2
  SubSystem1724/1(Block4700) -> Block4695/1
  Block4691/1 -> Block4694/1
  Block4694/1 -> SubSystem1724/2(Block4698)
  Block4694/2 -> SubSystem1728/2(Block4702)

== system lib_all/SubSystem1674/SubSystem1701/SubSystem1709/SubSystem1732 ==
  Block4706 : Inport
  Block4707 : Inport  Port=2
  Block4708 : EnablePort
  Block4709 : Demux  Outputs=2
  Block4710 : Mux  Inputs=2
  Block4711 : Sum  OutDataTypeStr=double  RndMeth=Zero  SaturateOnIntegerOverflow=on  InputSameDT=on
  SubSystem1733 : Subsystem  TreatAsAtomicUnit=on  mask(***ATC.DSRVEC***){bd_version=1.01.A, r0=DSM2, r1=-1, r2=, r3=, r4=, r5=, r6=, r7=, r8=, r9=, c0=, c1=, c2=, c3=, c4=, c5=, c6=, c7=, c8=, c9=}
      mask prompts: bd_version "Version"
      mask help: これはAutoCからのブロックです．編集禁止．
      settings: OpenFcn=warndlg('変更禁止!');
      mask Help: web(ui_blockhelpmng(gcb));
      mask Display: disp('ATC.DSRVEC')
  SubSystem1734 : Subsystem
  SubSystem1738 : Subsystem
  Block4720 : Outport
  wires:
  SubSystem1733/1(Output) -> Block4711/1
  Block4707/1 -> SubSystem1733/1(element), SubSystem1734/1(Block4712), SubSystem1738/1(Block4716)
  Block4710/1 -> Block4711/2
  Block4711/1 -> Block4720/1
  SubSystem1738/1(Block4719) -> Block4710/2
  SubSystem1734/1(Block4715) -> Block4710/1
  Block4709/2 -> SubSystem1738/2(Block4717)
  Block4709/1 -> SubSystem1734/2(Block4713)
  Block4706/1 -> Block4709/1

== system lib_all/SubSystem1742/SubSystem1745/SubSystem1746/SubSystem1747 ==
  note: ***編集禁止***
  In1 : Inport
  In2 : Inport  Port=2
  RO1 : RelationalOperator  Operator===  InputSameDT=on
  single1 : DataTypeConversion  OutDataTypeStr=single  SaturateOnIntegerOverflow=on  RndMeth=Zero
  single2 : DataTypeConversion  OutDataTypeStr=single  SaturateOnIntegerOverflow=on  RndMeth=Zero
  Out1 : Outport
  wires:
  RO1/1 -> Out1/1
  In2/1 -> single2/1
  In1/1 -> single1/1
  single1/1 -> RO1/1
  single2/1 -> RO1/2

== system lib_all/SubSystem1742/SubSystem1745/SubSystem1746/SubSystem1748 ==
  note: ***編集禁止***
  In1 : Inport
  In2 : Inport  Port=2
  RO1 : RelationalOperator  Operator===  InputSameDT=on
  single1 : DataTypeConversion  OutDataTypeStr=single  SaturateOnIntegerOverflow=on  RndMeth=Zero
  single2 : DataTypeConversion  OutDataTypeStr=single  SaturateOnIntegerOverflow=on  RndMeth=Zero
  Out1 : Outport
  wires:
  RO1/1 -> Out1/1
  In2/1 -> single2/1
  In1/1 -> single1/1
  single1/1 -> RO1/1
  single2/1 -> RO1/2

== system lib_all/SubSystem1742/SubSystem1745/SubSystem1746/SubSystem1749 ==
  Block4754 : Inport
  Block4755 : Inport  Port=2
  Block4756 : Inport  Port=3
  Block4757 : Sum  OutDataTypeStr=double  RndMeth=Zero  SaturateOnIntegerOverflow=on  InputSameDT=on
  Block4758 : Switch  Threshold=0.5  RndMeth=Zero  SaturateOnIntegerOverflow=on  InputSameDT=on
  SubSystem1750 : Subsystem
  Block4759 : Outport
  wires:
  Block4754/1 -> Block4757/1
  Block4758/1 -> Block4759/1
  Block4757/1 -> Block4758/1
  SubSystem1750/1(Out1) -> Block4758/2
  Block4756/1 -> SubSystem1750/2(In2)
  Block4755/1 -> Block4757/2, Block4758/3, SubSystem1750/1(In1)

== system lib_all/SubSystem1742/SubSystem1745/SubSystem1751/SubSystem1752 ==
  note: ***編集禁止***
  In1 : Inport
  In2 : Inport  Port=2
  LO1 : Logic
  RO1 : RelationalOperator  Operator=<  InputSameDT=on
  RO2 : RelationalOperator  Operator=~=  InputSameDT=on
  single1 : DataTypeConversion  OutDataTypeStr=single  SaturateOnIntegerOverflow=on  RndMeth=Zero
  single2 : DataTypeConversion  OutDataTypeStr=single  SaturateOnIntegerOverflow=on  RndMeth=Zero
  Out1 : Outport
  wires:
  In2/1 -> single2/1
  In1/1 -> single1/1
  single1/1 -> RO1/1, RO2/1
  single2/1 -> RO1/2, RO2/2
  RO1/1 -> LO1/1
  RO2/1 -> LO1/2
  LO1/1 -> Out1/1

== system lib_all/SubSystem1756/SubSystem1759/SubSystem1760/SubSystem1761 ==
  note: ***編集禁止***
  In1 : Inport
  In2 : Inport  Port=2
  RO1 : RelationalOperator  Operator===  InputSameDT=on
  single1 : DataTypeConversion  OutDataTypeStr=single  SaturateOnIntegerOverflow=on  RndMeth=Zero
  single2 : DataTypeConversion  OutDataTypeStr=single  SaturateOnIntegerOverflow=on  RndMeth=Zero
  Out1 : Outport
  wires:
  single2/1 -> RO1/2
  single1/1 -> RO1/1
  In1/1 -> single1/1
  In2/1 -> single2/1
  RO1/1 -> Out1/1

== system lib_all/SubSystem1756/SubSystem1759/SubSystem1760/SubSystem1762 ==
  note: ***編集禁止***
  In1 : Inport
  In2 : Inport  Port=2
  RO1 : RelationalOperator  Operator===  InputSameDT=on
  single1 : DataTypeConversion  OutDataTypeStr=single  SaturateOnIntegerOverflow=on  RndMeth=Zero
  single2 : DataTypeConversion  OutDataTypeStr=single  SaturateOnIntegerOverflow=on  RndMeth=Zero
  Out1 : Outport
  wires:
  single2/1 -> RO1/2
  single1/1 -> RO1/1
  In1/1 -> single1/1
  In2/1 -> single2/1
  RO1/1 -> Out1/1

== system lib_all/SubSystem1756/SubSystem1759/SubSystem1760/SubSystem1763 ==
  Block4813 : Inport
  Block4814 : Inport  Port=2
  Block4815 : Inport  Port=3
  Block4816 : Sum  OutDataTypeStr=double  RndMeth=Zero  SaturateOnIntegerOverflow=on  InputSameDT=on
  Block4817 : Switch  Threshold=0.5  RndMeth=Zero  SaturateOnIntegerOverflow=on  InputSameDT=on
  SubSystem1764 : Subsystem
  Block4818 : Outport
  wires:
  Block4814/1 -> SubSystem1764/1(In1), Block4817/3, Block4816/2
  Block4815/1 -> SubSystem1764/2(In2)
  SubSystem1764/1(Out1) -> Block4817/2
  Block4816/1 -> Block4817/1
  Block4817/1 -> Block4818/1
  Block4813/1 -> Block4816/1

== system lib_all/SubSystem1756/SubSystem1759/SubSystem1765/SubSystem1766 ==
  note: ***編集禁止***
  In1 : Inport
  In2 : Inport  Port=2
  LO1 : Logic
  RO1 : RelationalOperator  Operator=<  InputSameDT=on
  RO2 : RelationalOperator  Operator=~=  InputSameDT=on
  single1 : DataTypeConversion  OutDataTypeStr=single  SaturateOnIntegerOverflow=on  RndMeth=Zero
  single2 : DataTypeConversion  OutDataTypeStr=single  SaturateOnIntegerOverflow=on  RndMeth=Zero
  Out1 : Outport
  wires:
  LO1/1 -> Out1/1
  RO2/1 -> LO1/2
  RO1/1 -> LO1/1
  single2/1 -> RO2/2, RO1/2
  single1/1 -> RO2/1, RO1/1
  In1/1 -> single1/1
  In2/1 -> single2/1

== system lib_all/SubSystem176/SubSystem178/SubSystem182/SubSystem183 ==
  note: Text35
  Block413 : Inport
  Block414 : Inport  Port=2
  Block415 : Inport  Port=3
  Block416 : Inport  Port=4
  Block417 : Constant  Value=0  OutDataTypeStr=double  SampleTime=-1
  Block418 : Constant  OutDataTypeStr=double  SampleTime=-1
  Block419 : Constant  Value=0  OutDataTypeStr=double  SampleTime=-1
  Block420 : Gain  Gain=1/5  SaturateOnIntegerOverflow=on  OutDataTypeStr=Inherit: Same as input  ParamDataTypeStr=Inherit: Same as input
      settings: AttributesFormatString=Attribute100
  Block421 : Product  OutDataTypeStr=double  SaturateOnIntegerOverflow=on  RndMeth=Zero  InputSameDT=on
  Block422 : Sum  Inputs=-+  OutDataTypeStr=double  RndMeth=Zero  SaturateOnIntegerOverflow=on  InputSameDT=on
  Block423 : Sum  OutDataTypeStr=double  RndMeth=Zero  SaturateOnIntegerOverflow=on  InputSameDT=on
  Block424 : Sum  OutDataTypeStr=double  RndMeth=Zero  SaturateOnIntegerOverflow=on  InputSameDT=on
  Block425 : Switch  Threshold=0.5  RndMeth=Zero  SaturateOnIntegerOverflow=on  InputSameDT=on
  SubSystem185 : Subsystem
  SubSystem186 : Subsystem
  SubSystem192 : Subsystem
  Block534 : Outport
  wires:
  Block424/1 -> SubSystem192/3(Block455), SubSystem186/2(Block427)
  Block413/1 -> Block424/1
  Block420/1 -> Block423/2
  Block421/1 -> Block420/1
  Block418/1 -> Block424/2
  Block417/1 -> Block425/1
  Block425/1 -> Block534/1
  SubSystem185/1(Out1) -> Block425/2
  Block419/1 -> SubSystem185/2(In2)
  Block415/1 -> SubSystem186/3(Block428)
  Block423/1 -> SubSystem185/1(In1), Block425/3
  SubSystem186/2(Block452) -> Block421/2
  Block422/1 -> Block421/1  "SigLabel11"
  SubSystem192/2(Block533) -> Block422/2
  SubSystem192/1(Block532) -> Block422/1, Block423/1
  Block416/1 -> SubSystem192/2(Block454)
  SubSystem186/1(Block451) -> SubSystem192/1(Block453)
  Block414/1 -> SubSystem186/1(Block426)
